simulink model "PrkgClimaActvMgr"  (R2022b)
settings: Solver=FixedStepDiscrete  StartTime=0.0  StopTime=10.0  FixedStep=0.2  RelTol=1e-3
(part 1 of 4)
settings Model: Creator=blue.ge  LastModifiedBy=blue.ge
settings DataIOCC: SaveFormat=StructureWithTime
settings OptimizationCC: DefaultParameterBehavior=Inlined  EnhancedBackFolding=on  PassReuseOutputArgsAs=Individual arguments  ZeroExternalMemoryAtStartup=off  ZeroInternalMemoryAtStartup=off  OptimizeBlockOrder=speed  DifferentSizesBufferReuse=on  OptimizationCustomize=off  EfficientTunableParamExpr=on
settings DebuggingCC: ParameterTunabilityLossMsg=error
settings ModelReferenceCC: ModelReferenceNumInstancesAllowed=Single
settings SFSimCC: MATLABDynamicMemAlloc=off
settings RTWCC: Description=AUTOSAR  SystemTargetFile=autosar.tlc  GenCodeOnly=on  TemplateMakefile=ert_default_tmf
settings CodeAppCC: ForceParamTrailComments=on  IgnoreCustomStorageClasses=off  OperatorAnnotations=on  SimulinkDataObjDesc=on  SFDataObjDesc=on  InsertBlockDesc=on
settings STFCustomTargetCC: TargetLangStandard=C99 (ISO)  MultiwordLength=256  ParMdlRefBuildCompliant=on  CompOptLevelCompliant=on  IncludeMdlTerminateFcn=off  CombineOutputUpdateFcns=on  SuppressErrorStatus=on  ERTCustomFileBanners=on  SupportNonFinite=off  SupportComplex=off  SupportContinuousTime=off  SupportNonInlinedSFcns=off  RemoveResetFunc=on  MATLABClassNameForMDSCustomization=Simulink.SoftwareTarget.ERTCustomization  AutosarCompliant=on  UseToolchainInfoCompliant=on  LookupTableObjectStructAxisOrder=2,1,3,4,...  LUTObjectStructOrderEvenSpacing=Size,Table,Breakpoints  SystemTargetFile=autosar.tlc
settings ERTTargetCC: TargetLangStandard=C99 (ISO)  ConcurrentExecutionCompliant=off  IncludeMdlTerminateFcn=off  SuppressErrorStatus=on  SupportNonFinite=off  SupportComplex=off  ModelStepFunctionPrototypeControlCompliant=off  CPPClassGenCompliant=off  AutosarCompliant=on  LookupTableObjectStructAxisOrder=2,1,3,4,...  LUTObjectStructOrderEvenSpacing=Size,Table,Breakpoints  InlinedParameterPlacement=NonHierarchical  ERTCustomFileTemplate=example_file_process.tlc

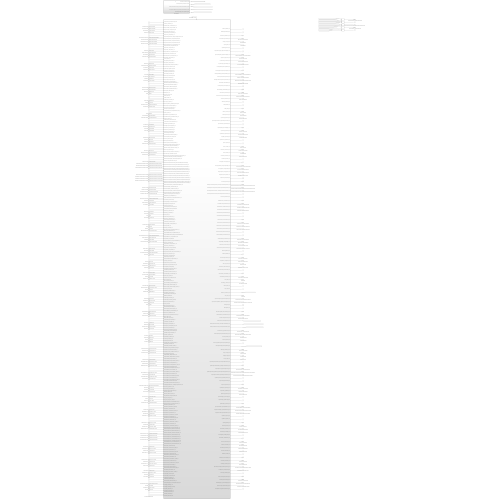
[thumbnail: Simulink's stored preview of the top-level diagram]
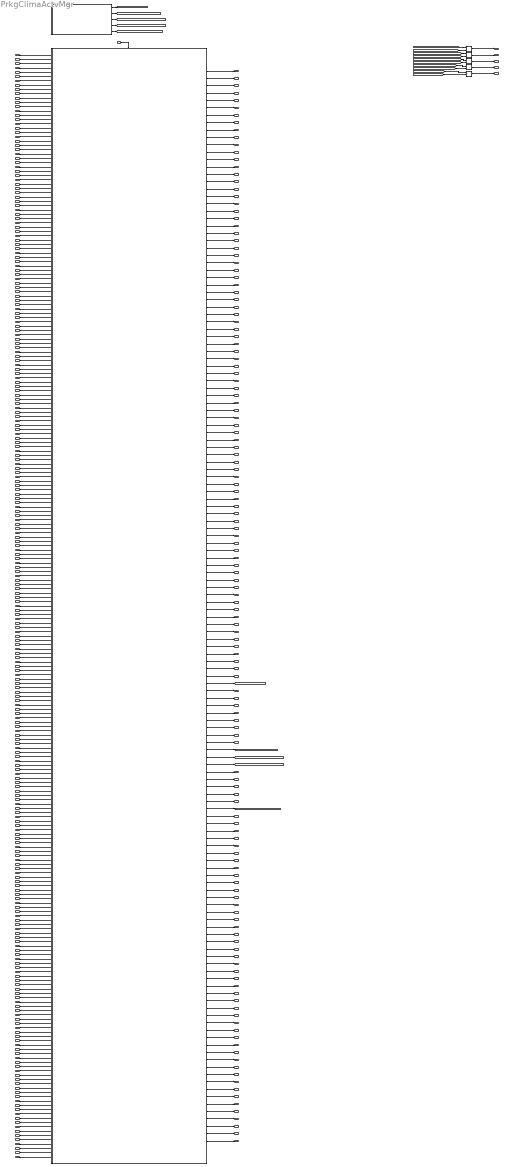
== system PrkgClimaActvMgr ==
  PrkgClimaActvMgr_Runnable : Inport  SampleTime=0.2  OutputFunctionCall=on
  ActvRqrdForPostPrkgClima_ActvRqrdForPostPrkgClima : Inport  Port=2  OutDataTypeStr=Boolean  PortDimensions=1
  CarTiIntForClima_CarTiIntForClima : Inport  Port=3  OutDataTypeStr=CarTiGlb  PortDimensions=1
  CdsClimaOnReqfromExsrc_CdsClimaOnReqfromExsrc : Inport  Port=4  OutDataTypeStr=Enum: OnOff1  PortDimensions=1
  ClimaCfgForClimaCtrlMan1_ClimaCfgForClimaCtrlMan1 : Inport  Port=5  OutDataTypeStr=Boolean  PortDimensions=1
  ClimaCfgForCmptmtClng_ClimaCfgForCmptmtClng : Inport  Port=6  OutDataTypeStr=Boolean  PortDimensions=1
  ClimaCfgForFoldCSD_ClimaCfgForFoldCSD : Inport  Port=7  OutDataTypeStr=Boolean  PortDimensions=1
  ClimaCfgForNrOfTSetg1_ClimaCfgForNrOfTSetg1 : Inport  Port=8  OutDataTypeStr=Enum: ClimaCfgForNrOfTSetg1  PortDimensions=1
  ClimaCfgForPreClng1_ClimaCfgForPreClng1 : Inport  Port=9  OutDataTypeStr=Boolean  PortDimensions=1
  ClimaCfgForSeatHeatgForRowFirstPrsnt1_ClimaCfgForSeatHeatgForRowFirstPrsnt1 : Inport  Port=10  OutDataTypeStr=Boolean  PortDimensions=1
  ClimaCfgForSeatHeatgForRowSec_ClimaCfgForSeatHeatgForRowSec : Inport  Port=11  OutDataTypeStr=Enum: CarCfgForSeatHeatgForRowSecGly  PortDimensions=1
  ClimaCfgForSeatVentnForRowFirst_ClimaCfgForSeatVentnForRowFirst : Inport  Port=12  OutDataTypeStr=Enum: CarCfgForSeatVentnForRowFirst1  PortDimensions=1
  ClimaCfgForSeatVentnForRowSec_ClimaCfgForSeatVentnForRowSec : Inport  Port=13  OutDataTypeStr=Enum: CarCfgForSeatVentnForRowSecGly  PortDimensions=1
  ClimaCfgForSteerWhlHeatgPrsnt1_ClimaCfgForSteerWhlHeatgPrsnt1 : Inport  Port=14  OutDataTypeStr=Boolean  PortDimensions=1
  ClimaCfgForSteerWhlLeSide1_ClimaCfgForSteerWhlLeSide1 : Inport  Port=15  OutDataTypeStr=Boolean  PortDimensions=1
  ClimaCfgForVehElec1_ClimaCfgForVehElec1 : Inport  Port=16  OutDataTypeStr=Boolean  PortDimensions=1
  ClimaCfgForVehHyb1_ClimaCfgForVehHyb1 : Inport  Port=17  OutDataTypeStr=Boolean  PortDimensions=1
  ClimaCfgForWindHeatrPrsnt1_ClimaCfgForWindHeatrPrsnt1 : Inport  Port=18  OutDataTypeStr=Boolean  PortDimensions=1
  ClimaOvrHeatProWarnIni_ClimaOvrHeatProWarnIni : Inport  Port=19  OutDataTypeStr=Enum: ClimaOvrheatProWarnSts  PortDimensions=1
  ClimaOvrHeatReqFromExrsc_ClimaOvrHeatReqFromExrsc : Inport  Port=20  OutDataTypeStr=Enum: PreCdngReqFromExtSrc  PortDimensions=1
  ClimaOvrHeatStrtReq_ClimaOvrHeatStrtReq : Inport  Port=21  OutDataTypeStr=Boolean  PortDimensions=1
  CmptmtClsd_CmptmtClsd : Inport  Port=22  OutDataTypeStr=Boolean  PortDimensions=1
  CSDUsgChgeOffReq_CSDUsgChgeOffReq : Inport  Port=23  OutDataTypeStr=Boolean  PortDimensions=1
  CSDUsgChgeOnReq_CSDUsgChgeOnReq : Inport  Port=24  OutDataTypeStr=Boolean  PortDimensions=1
  DefrstHeatgForPrkgClimaEnad_DefrstHeatgForPrkgClimaEnad : Inport  Port=25  OutDataTypeStr=Boolean  PortDimensions=1
  DoorOpenStsForClima_DoorOpenStsForClima : Inport  Port=26  OutDataTypeStr=Boolean  PortDimensions=1
  EgyAllwdForPrkgClima_EgyAllwdForPrkgClima : Inport  Port=27  OutDataTypeStr=Boolean  PortDimensions=1
  EgyPrioForPrkgClima_EgyPrioForPrkgClima : Inport  Port=28  OutDataTypeStr=Enum: EgyPrio  PortDimensions=1
  ErsCmdByKeyStrtReq_ErsCmdByKeyStrtReq : Inport  Port=29  OutDataTypeStr=Enum: OnOff1  PortDimensions=1
  ErsRunTimelStrtReq_ErsRunTimelStrtReq : Inport  Port=30  OutDataTypeStr=Ti39  PortDimensions=1
  FacForRtForPrkgClima_FacForRtForPrkgClima : Inport  Port=31  OutDataTypeStr=Fac30  PortDimensions=1
  FrntHvacBlowerSts_FrntHvacBlowerSts : Inport  Port=32  OutDataTypeStr=Enum: HvacFanSts  PortDimensions=1
  HeatrTypForPrkgClima_HeatrTypForPrkgClima : Inport  Port=33  OutDataTypeStr=Enum: HeatrTyp  PortDimensions=1
  HeatUpRqrdForPrkgClima_HeatUpRqrdForPrkgClima : Inport  Port=34  OutDataTypeStr=Boolean  PortDimensions=1
  HMIExtVentFanVolReqStrtReq_HMIExtVentFanVolReqStrtReq : Inport  Port=35  OutDataTypeStr=Enum: FanVolReq  PortDimensions=1
  HMIExtVentonoffReqStrtReq_HMIExtVentonoffReqStrtReq : Inport  Port=36  OutDataTypeStr=Enum: OnOffNoReq  PortDimensions=1
  HMIExtVentTSpReqStrtReq_HMIExtVentTSpReqStrtReq : Inport  Port=37  OutDataTypeStr=Enum: TSpReq  PortDimensions=1
  HMIExtVentTStsReqStrtReq_HMIExtVentTStsReqStrtReq : Inport  Port=38  OutMin=-256  OutMax=255.9  OutDataTypeStr=T2  PortDimensions=1
  HvBattForPrkgClima_HvBattForPrkgClima : Inport  Port=39  OutDataTypeStr=Boolean  PortDimensions=1
  HvBattSoc_HvBattSoc : Inport  Port=40  OutDataTypeStr=Perc7  PortDimensions=1
  HvSysCnctWoU_HvSysCnctWoU : Inport  Port=41  OutDataTypeStr=Boolean  PortDimensions=1
  HvSysWoU_HvSysWoU : Inport  Port=42  OutDataTypeStr=Boolean  PortDimensions=1
  HvSysRlySts_HvSysRlySts : Inport  Port=43  OutDataTypeStr=Bus: HvSysRlySts  PortDimensions=1  BusOutputAsStruct=on
  IntCo2MontrStrtReq_IntCo2MontrStrtReq : Inport  Port=44  OutDataTypeStr=Boolean  PortDimensions=1
  LockgCenSts_Ngcc_LockgCenSts : Inport  Port=45  OutDataTypeStr=Bus: LockgCenSts3  PortDimensions=1  BusOutputAsStruct=on
  MemReadForPrkgClimaActvMgr_MemReadForPrkgClimaActvMgr : Inport  Port=46  OutDataTypeStr=Bus: MemRecForPrkgClimaActvMgr  PortDimensions=1  BusOutputAsStruct=on
  MemReqStsForPrkgClima_MemReqStsForPrkgClima : Inport  Port=47  OutDataTypeStr=Enum: MemReqSts  PortDimensions=1
  OffForTLoForPostCdng_OffForTLoForPostCdng : Inport  Port=48  OutDataTypeStr=Boolean  PortDimensions=1
  OffRqrdForPrkgClima_OffRqrdForPrkgClima : Inport  Port=49  OutDataTypeStr=Boolean  PortDimensions=1
  PrePrkgClimaNotAvlForHvSysWoU_PrePrkgClimaNotAvlForHvSysWoU : Inport  Port=50  OutDataTypeStr=Boolean  PortDimensions=1
  PrkgCDSAvl_PrkgCDSAvl : Inport  Port=51  OutDataTypeStr=Boolean  PortDimensions=1
  PrkgClimaAllwdForBattULo_PrkgClimaAllwdForBattULo : Inport  Port=52  OutDataTypeStr=Boolean  PortDimensions=1
  PrkgClimaAllwdForSwtHeatrTyp_PrkgClimaAllwdForSwtHeatrTyp : Inport  Port=53  OutDataTypeStr=Boolean  PortDimensions=1
  PrkgHeatgActv_PrkgHeatgActv : Inport  Port=54  OutDataTypeStr=Boolean  PortDimensions=1
  PwrAllwdForHvacFanFrnt_PwrAllwdForHvacFanFrnt : Inport  Port=55  OutDataTypeStr=Pwr33  PortDimensions=1
  PwrCnsDesForHvacFanFrnt_PwrCnsDesForHvacFanFrnt : Inport  Port=56  OutDataTypeStr=Pwr33  PortDimensions=1
  RemClimaallExsrcReq_RemClimaallExsrcReq : Inport  Port=57  OutDataTypeStr=UInt32  PortDimensions=1
  RemClimaEgyExsrcReq_RemClimaEgyExsrcReq : Inport  Port=58  OutDataTypeStr=UInt32  PortDimensions=1
  RemClimaTmrStrtReq_RemClimaTmrStrtReq : Inport  Port=59  OutDataTypeStr=Ti33  PortDimensions=1
  RemStrtExtnTiStrtReq_RemStrtExtnTiStrtReq : Inport  Port=60  OutDataTypeStr=Ti39  PortDimensions=1
  RemStrtHvCtrlStrtReq_RemStrtHvCtrlStrtReq : Inport  Port=61  OutDataTypeStr=Enum: ErsCmd  PortDimensions=1
  RemVentReqStrtReq_RemVentReqStrtReq : Inport  Port=62  OutDataTypeStr=Boolean  PortDimensions=1
  RtCmftForImdtPrePrkgClima_RtCmftForImdtPrePrkgClima : Inport  Port=63  OutDataTypeStr=Ti33  PortDimensions=1
  RtCmftForPostPrkgClima_RtCmftForPostPrkgClima : Inport  Port=64  OutDataTypeStr=Ti33  PortDimensions=1
  RtCmftForPreClng_RtCmftForPreClng : Inport  Port=65  OutDataTypeStr=Ti33  PortDimensions=1
  RtSdlForPrkgClima_RtSdlForPrkgClima : Inport  Port=66  OutDataTypeStr=Ti33  PortDimensions=1
  SeatHeatForPrkgClimaEnad_SeatHeatForPrkgClimaEnad : Inport  Port=67  OutDataTypeStr=Enum: SeatHeatDurgClimaEnad  PortDimensions=1
  SteerWhlHeatgForPrkgClimaEnad_SteerWhlHeatgForPrkgClimaEnad : Inport  Port=68  OutDataTypeStr=Boolean  PortDimensions=1
  StrtRemSts1WdStrtRemSts_Ngcc_StrtRemSts1WdStrtRemSts : Inport  Port=69  OutDataTypeStr=Enum: StrtRemSts1  PortDimensions=1
  StrtRqrdForDirForPrePrkgClima_StrtRqrdForDirForPrePrkgClima : Inport  Port=70  OutDataTypeStr=Boolean  PortDimensions=1
  StrtRqrdForPreClng_StrtRqrdForPreClng : Inport  Port=71  OutDataTypeStr=Boolean  PortDimensions=1
  StrtRqrdForTmrForPrePrkgClima_StrtRqrdForTmrForPrePrkgClima : Inport  Port=72  OutDataTypeStr=Boolean  PortDimensions=1
  SuspStrtAllwdForPrkgClima_SuspStrtAllwdForPrkgClima : Inport  Port=73  OutDataTypeStr=Boolean  PortDimensions=1
  TelmBookClimaReqHmiCmptmtTSpSpclStrtReq_TelmBookClimaReqHmiCmptmtTSpSpclStrtReq : Inport  Port=74  OutDataTypeStr=Enum: NormalLowHigh  PortDimensions=1
  TelmBookClimaReqOnOffNoReqStrtReq_TelmBookClimaReqOnOffNoReqStrtReq : Inport  Port=75  OutDataTypeStr=Enum: NoReqOnOff  PortDimensions=1
  TelmBookClimaReqTempRangeStrtReq_TelmBookClimaReqTempRangeStrtReq : Inport  Port=76  OutDataTypeStr=TempRange  PortDimensions=1
  TelmBookClimaTmrStrtReq_TelmBookClimaTmrStrtReq : Inport  Port=77  OutDataTypeStr=Ti33  PortDimensions=1
  TelmBookSeatHeatClimalReqDrvHeatClimaLvlStrtReq_TelmBookSeatHeatClimalReqDrvHeatClimaLvlStrtReq : Inport  Port=78  OutDataTypeStr=Enum: TelmSeatClimaLvl  PortDimensions=1
  TelmBookSeatHeatClimalReqPassHeatClimaLvlStrtReq_TelmBookSeatHeatClimalReqPassHeatClimaLvlStrtReq : Inport  Port=79  OutDataTypeStr=Enum: TelmSeatClimaLvl  PortDimensions=1
  TelmBookSeatHeatClimalReqSecLeHeatClimaLvlStrtReq_TelmBookSeatHeatClimalReqSecLeHeatClimaLvlStrtReq : Inport  Port=80  OutDataTypeStr=Enum: TelmSeatClimaLvl  PortDimensions=1
  TelmBookSeatHeatClimalReqSecRiHeatClimaLvlStrtReq_TelmBookSeatHeatClimalReqSecRiHeatClimaLvlStrtReq : Inport  Port=81  OutDataTypeStr=Enum: TelmSeatClimaLvl  PortDimensions=1
  TelmBookSeatHeatClimalReqThrdLeHeatClimaLvlStrtReq_TelmBookSeatHeatClimalReqThrdLeHeatClimaLvlStrtReq : Inport  Port=82  OutDataTypeStr=Enum: TelmSeatClimaLvl  PortDimensions=1
  TelmBookSeatHeatClimalReqThrdRiHeatClimaLvlStrtReq_TelmBookSeatHeatClimalReqThrdRiHeatClimaLvlStrtReq : Inport  Port=83  OutDataTypeStr=Enum: TelmSeatClimaLvl  PortDimensions=1
  TelmBookSeatVentnClimalReqDrvVentnClimaLvlStrtReq_TelmBookSeatVentnClimalReqDrvVentnClimaLvlStrtReq : Inport  Port=84  OutDataTypeStr=Enum: TelmSeatClimaLvl  PortDimensions=1
  TelmBookSeatVentnClimalReqPassVentnClimaLvlStrtReq_TelmBookSeatVentnClimalReqPassVentnClimaLvlStrtReq : Inport  Port=85  OutDataTypeStr=Enum: TelmSeatClimaLvl  PortDimensions=1
  TelmBookSeatVentnClimalReqSecLeVentnClimaLvlStrtReq_TelmBookSeatVentnClimalReqSecLeVentnClimaLvlStrtReq : Inport  Port=86  OutDataTypeStr=Enum: TelmSeatClimaLvl  PortDimensions=1
  TelmBookSeatVentnClimalReqSecRiVentnClimaLvlStrtReq_TelmBookSeatVentnClimalReqSecRiVentnClimaLvlStrtReq : Inport  Port=87  OutDataTypeStr=Enum: TelmSeatClimaLvl  PortDimensions=1
  TelmBookSeatVentnClimalReqThrdLeVentnClimaLvlStrtReq_TelmBookSeatVentnClimalReqThrdLeVentnClimaLvlStrtReq : Inport  Port=88  OutDataTypeStr=Enum: TelmSeatClimaLvl  PortDimensions=1
  TelmBookSeatVentnClimalReqThrdRiVentnClimaLvlStrtReq_TelmBookSeatVentnClimalReqThrdRiVentnClimaLvlStrtReq : Inport  Port=89  OutDataTypeStr=Enum: TelmSeatClimaLvl  PortDimensions=1
  TelmBookSteerWhlHeatClimalReqStrtReq_TelmBookSteerWhlHeatClimalReqStrtReq : Inport  Port=90  OutDataTypeStr=Enum: OnOff1  PortDimensions=1
  TelmClimaExtVentnLvlStrtReq_TelmClimaExtVentnLvlStrtReq : Inport  Port=91  OutDataTypeStr=Enum: HmiHvacFanLvl  PortDimensions=1
  TelmClimaExtVentnReqStrtReq_TelmClimaExtVentnReqStrtReq : Inport  Port=92  OutDataTypeStr=Enum: OnOffNoReq  PortDimensions=1
  TelmClimaExtVentnTempRangeStrtReq_TelmClimaExtVentnTempRangeStrtReq : Inport  Port=93  OutMin=-256  OutMax=255.9  OutDataTypeStr=T2  PortDimensions=1
  TelmClimaExtVentnTSpSpclStrtReq_TelmClimaExtVentnTSpSpclStrtReq : Inport  Port=94  OutDataTypeStr=Enum: HmiCmptmtTSpSpcl  PortDimensions=1
  UpdRtTotForTmrForPrePrkgClima_UpdRtTotForTmrForPrePrkgClima : Inport  Port=95  OutDataTypeStr=Boolean  PortDimensions=1
  VehModMngtGlbSafe1_Ngcc_VehModMngtGlbSafe1 : Inport  Port=96  OutDataTypeStr=Bus: VehModMngtGlbSafe1  PortDimensions=1  BusOutputAsStruct=on
  VFCBodyPreClimatisationHmiHumCtrl_VFCBodyPreClimatisationHmiHumCtrl : Inport  Port=97  OutDataTypeStr=Boolean  PortDimensions=1
  VFCHmiClimaCtrlAct_VFCHmiClimaCtrlAct : Inport  Port=98  OutDataTypeStr=Enum: OnOff1  PortDimensions=1
  VFCPrkDrvgClimabyFragAct_VFCPrkDrvgClimabyFragAct : Inport  Port=99  OutDataTypeStr=Boolean  PortDimensions=1
  WarnForEgyAllwdLimd_WarnForEgyAllwdLimd : Inport  Port=100  OutDataTypeStr=Boolean  PortDimensions=1
  WarnForHybWoU_WarnForHybWoU : Inport  Port=101  OutDataTypeStr=Boolean  PortDimensions=1
  WarnForPreCdngFctNotAvl_WarnForPreCdngFctNotAvl : Inport  Port=102  OutDataTypeStr=Boolean  PortDimensions=1
  WarnForPrkgClimaNotAllwd_WarnForPrkgClimaNotAllwd : Inport  Port=103  OutDataTypeStr=Enum: ClimaWarn  PortDimensions=1
  WindIceDetdFrnt_Ngcc_WindIceDetdFrnt : Inport  Port=104  OutDataTypeStr=Enum: OnOff1  PortDimensions=1
  WindIceDetdRe_Ngcc_WindIceDetdRe : Inport  Port=105  OutDataTypeStr=Enum: OnOff1  PortDimensions=1
  AirCdnAvl_AirCdnAvl : Inport  Port=106  OutDataTypeStr=Boolean  PortDimensions=1
  AmbTFildForClimaNgcc_AmbTFildForClima : Inport  Port=107  OutDataTypeStr=T30  PortDimensions=1
  OffRqrdForRemClima_OffRqrdForRemClima : Inport  Port=108  OutDataTypeStr=Boolean  PortDimensions=1
  RemClimaTempStrtReq_RemClimaTempStrtReq : Inport  Port=109  OutMin=-256  OutMax=255.9  OutDataTypeStr=T2  PortDimensions=1
  VFCAutDemstOperSts_VFCAutDemstOperSts : Inport  Port=110  OutDataTypeStr=Boolean  PortDimensions=1
  VFCPrkDrvgClimabyPM25_VFCPrkDrvgClimabyPM25 : Inport  Port=111  OutDataTypeStr=Boolean  PortDimensions=1
  AirCdnAvlRe_AirCdnAvlRe : Inport  Port=112  OutDataTypeStr=Boolean  PortDimensions=1
  AirSrcStsFbNgcc_AirSrcStsFbNgcc : Inport  Port=113  OutMin=0  OutMax=3  OutDataTypeStr=UInt2  PortDimensions=1
  BookTrvlClimaActvReqfromExsrc_BookTrvlClimaActvReqfromExsrc : Inport  Port=114  OutDataTypeStr=Enum: OnOffNoReq  PortDimensions=1
  CCMWakeupFunction_CCMWakeupFunction : Inport  Port=115  OutDataTypeStr=UInt8  PortDimensions=1
  ClimaCfgFor3RDROWSEATHEAT_ClimaCfgFor3RDROWSEATHEAT : Inport  Port=116  OutDataTypeStr=UInt8  PortDimensions=1
  ClimaCfgFor3RDROWSEATVENTILATED_ClimaCfgFor3RDROWSEATVENTILATED : Inport  Port=117  OutDataTypeStr=UInt8  PortDimensions=1
  ClimaCfgForAirMtrlSnsrPrsnt1_ClimaCfgForAirMtrlSnsrPrsnt1 : Inport  Port=118  OutDataTypeStr=Enum: CarCfgForAirMtrlSnsrGly  PortDimensions=1
  ClimaCfgForBRAND_ClimaCfgForBRAND : Inport  Port=119  OutDataTypeStr=Enum: ClimaCfgForBRAND  PortDimensions=1
  ClimaCfgForDrvrSeatVentWthSmf2_ClimaCfgForDrvrSeatVentWthSmf2 : Inport  Port=120  OutDataTypeStr=UInt8  PortDimensions=1
  ClimaCfgForErev1_ClimaCfgForErev1 : Inport  Port=121  OutDataTypeStr=Boolean  PortDimensions=1
  ClimaCfgForHvacRePrsnt1_ClimaCfgForHvacRePrsnt1 : Inport  Port=122  OutDataTypeStr=Enum: CarCfgForHvacRe  PortDimensions=1
  ClimaCfgForHvacTyp_ClimaCfgForHvacTyp : Inport  Port=123  OutDataTypeStr=Enum: CarCfgForHvacTyp2  PortDimensions=1
  ClimaCfgForLifeDetection_ClimaCfgForLifeDetection : Inport  Port=124  OutDataTypeStr=Enum: LifeDetection  PortDimensions=1
  ClimaCfgForMARKET_ClimaCfgForMARKET : Inport  Port=125  OutDataTypeStr=UInt8  PortDimensions=1
  ClimaCfgForPassSeatVentWthSmf2_ClimaCfgForPassSeatVentWthSmf2 : Inport  Port=126  OutDataTypeStr=UInt8  PortDimensions=1
  ClimaCfgForPrpsnTyp_ClimaCfgForPrpsnTyp : Inport  Port=127  OutDataTypeStr=Enum: CarCfgForPrpsnTypGly  PortDimensions=1
  ClimaCfgForRoofHatch_ClimaCfgForRoofHatch : Inport  Port=128  OutDataTypeStr=Enum: CarCfgForRoofHatch  PortDimensions=1
  ClimaCfgForVehTyp_ClimaCfgForVehTyp : Inport  Port=129  OutDataTypeStr=Enum: CarCfgForVehTyp3  PortDimensions=1
  ClimaCfgReqVersionReplace_ClimaCfgReqVersionReplace : Inport  Port=130  OutDataTypeStr=UInt8  PortDimensions=1
  CmptmtClngActv_CmptmtClngActv : Inport  Port=131  OutDataTypeStr=Enum: CmptmtClngActv  PortDimensions=1
  CmptmtOpenStsForClima_CmptmtOpenStsForClima : Inport  Port=132  OutDataTypeStr=Boolean  PortDimensions=1
  DoorStsWithOutTrForClima_DoorStsWithOutTrForClima : Inport  Port=133  OutDataTypeStr=Boolean  PortDimensions=1
  DrvrSeatHeatgLvlSts_DrvrSeatHeatgLvlSts : Inport  Port=134  OutDataTypeStr=Enum: SeatClimaLvl  PortDimensions=1
  DrvrSeatHeatgLvlSts_DrvrSeatHeatgLvlSts_IsUpdated : Inport  Port=135  OutDataTypeStr=boolean  PortDimensions=1
  DrvrSeatVentnLvlSts_DrvrSeatVentnLvlSts : Inport  Port=136  OutDataTypeStr=Enum: SeatClimaLvl  PortDimensions=1
  DrvrSeatVentnLvlSts_DrvrSeatVentnLvlSts_IsUpdated : Inport  Port=137  OutDataTypeStr=boolean  PortDimensions=1
  DrvrSeatVentnLvlSts2Ngcc_DrvrSeatVentnLvlSts2Ngcc : Inport  Port=138  OutDataTypeStr=Enum: SeatClimaLvl  PortDimensions=1
  EngSt1WdSts_Ngcc_EngSt1WdSts : Inport  Port=139  OutDataTypeStr=Enum: EngSt1  PortDimensions=1
  ErsCmdByKeyFromExsrc_ErsCmdByKeyFromExsrc : Inport  Port=140  OutDataTypeStr=Enum: ErsCmd  PortDimensions=1
  GearLvrIndcn_GearLvrIndcn : Inport  Port=141  OutDataTypeStr=Enum: GearLvrIndcn2  PortDimensions=1
  GearLvrIndcn_GearLvrIndcn_IsUpdated : Inport  Port=142  OutDataTypeStr=boolean  PortDimensions=1
  HighTempCleanHmiReqNgcc_HighTempCleanHmiReqNgcc : Inport  Port=143  OutDataTypeStr=Enum: NoReqOnOff  PortDimensions=1
  HMIAirCdnrAutDrySetNgcc_HMIAirCdnrAutDrySetNgcc : Inport  Port=144  OutDataTypeStr=Enum: OnOff1  PortDimensions=1
  HMIAirCdnrAutDrySetNgcc_HMIAirCdnrAutDrySetNgcc_IsUpdated : Inport  Port=145  OutDataTypeStr=boolean  PortDimensions=1
  HmiCmptmtTSp_HmiCmptmtTSp : Inport  Port=146  OutDataTypeStr=Bus: HmiCmptmtTSp  PortDimensions=1  BusOutputAsStruct=on
  HMILBRACStrtSetNgcc_HMILBRACStrtSetNgcc : Inport  Port=147  OutDataTypeStr=Enum: OnOff1  PortDimensions=1
  HmiParkVentSetNgcc_HmiParkVentSetNgcc : Inport  Port=148  OutDataTypeStr=Enum: OnOff1  PortDimensions=1
  HmiParkVentTmrSetNgcc_HmiParkVentTmrSetNgcc : Inport  Port=149  OutMin=0  OutMax=31  OutDataTypeStr=TimeHr5  PortDimensions=1
  HvBattMntnActv_HvBattMntnActv : Inport  Port=150  OutDataTypeStr=Boolean  PortDimensions=1
  HvCooltHeatrStsSig_HvCooltHeatrStsSig : Inport  Port=151  OutDataTypeStr=Enum: HvCooltHeatrSts  PortDimensions=1
  HvCooltHeatrStsSig_HvCooltHeatrStsSig_IsUpdated : Inport  Port=152  OutDataTypeStr=boolean  PortDimensions=1
  IntPm25VluFrmClima_IntPm25VluFrmClima : Inport  Port=153  OutDataTypeStr=UInt10  PortDimensions=1
  LDACState_LDACState : Inport  Port=154  OutDataTypeStr=Boolean  PortDimensions=1
  MemBookTrvlClimaTi_MemBookTrvlClimaTi : Inport  Port=155  OutDataTypeStr=Mins2  PortDimensions=1
  MemReadForBlwAftRun_MemReadForBlwAftRun : Inport  Port=156  OutDataTypeStr=Bus: MemRecForBlwAftRun  PortDimensions=1  BusOutputAsStruct=on
  MemReadForHighTempCln_MemReadForHighTempCln : Inport  Port=157  OutDataTypeStr=Bus: MemRecForHighTempCln  PortDimensions=1  BusOutputAsStruct=on
  MemReadForHMISetEngReq_MemReadForHMISetEngReq : Inport  Port=158  OutDataTypeStr=Bus: MemRecForHMISetEngReq  PortDimensions=1  BusOutputAsStruct=on
  MemReadForParkVent_MemReadForParkVent : Inport  Port=159  OutDataTypeStr=Bus: MemRecForParkVent  PortDimensions=1  BusOutputAsStruct=on
  MemReadForParkVentActvCnt_MemReadForParkVentActvCnt : Inport  Port=160  OutDataTypeStr=Bus: MemRecForParkVentCnt  PortDimensions=1  BusOutputAsStruct=on
  OnBdChrgrSt_OnBdChrgrSt : Inport  Port=161  OutDataTypeStr=Enum: ChrgrSts1  PortDimensions=1
  OnBdChrgrSt_OnBdChrgrSt_IsUpdated : Inport  Port=162  OutDataTypeStr=boolean  PortDimensions=1
  OxygenSplyAvlStsNgcc_OxygenSplyAvlStsNgcc : Inport  Port=163  OutDataTypeStr=Enum: OxygenSplyAvlSts  PortDimensions=1
  OxygenSplyErrNgcc_OxygenSplyErrNgcc : Inport  Port=164  OutDataTypeStr=Enum: Err1  PortDimensions=1
  PassSeatHeatgLvlSts_PassSeatHeatgLvlSts : Inport  Port=165  OutDataTypeStr=Enum: SeatClimaLvl  PortDimensions=1
  PassSeatHeatgLvlSts_PassSeatHeatgLvlSts_IsUpdated : Inport  Port=166  OutDataTypeStr=boolean  PortDimensions=1
  PassSeatVentnLvlSts_PassSeatVentnLvlSts : Inport  Port=167  OutDataTypeStr=Enum: SeatClimaLvl  PortDimensions=1
  PassSeatVentnLvlSts_PassSeatVentnLvlSts_IsUpdated : Inport  Port=168  OutDataTypeStr=boolean  PortDimensions=1
  PassSeatVentnLvlSts2Ngcc_PassSeatVentnLvlSts2Ngcc : Inport  Port=169  OutDataTypeStr=Enum: SeatClimaLvl  PortDimensions=1
  PrkgClimaReqExcpSeat_PrkgClimaReqExcpSeat : Inport  Port=170  OutDataTypeStr=Boolean  PortDimensions=1
  PrkgClimaReqPrio_PrkgClimaReqPrio : Inport  Port=171  OutDataTypeStr=Boolean  PortDimensions=1
  PrkgOGMAirSrcReq_PrkgOGMAirSrcReq : Inport  Port=172  OutDataTypeStr=Boolean  PortDimensions=1
  PrvntWinActvReq_PrvntWinActvReq : Inport  Port=173  OutDataTypeStr=Boolean  PortDimensions=1
  ReHvacBlowerSts_ReHvacBlowerSts : Inport  Port=174  OutDataTypeStr=Enum: HvacFanSts  PortDimensions=1
  RemClimaEgyExsrcReqPlus_RemClimaEgyExsrcReqPlus : Inport  Port=175  OutDataTypeStr=UInt32  PortDimensions=1
  RemClimaHVReqFb_RemClimaHVReqFb : Inport  Port=176  OutDataTypeStr=Enum: HVReqFb  PortDimensions=1
  RemClimaHVReqFb_RemClimaHVReqFb_IsUpdated : Inport  Port=177  OutDataTypeStr=boolean  PortDimensions=1
  RemClimaWarnForCmptmtClng_RemClimaWarnForCmptmtClng : Inport  Port=178  OutDataTypeStr=Enum: ClimaWarn  PortDimensions=1
  RemClimaWarnForOpenGlb_RemClimaWarnForOpenGlb : Inport  Port=179  OutDataTypeStr=Enum: ClimaWarn  PortDimensions=1
  RemClimaWarnForUnLckClima_RemClimaWarnForUnLckClima : Inport  Port=180  OutDataTypeStr=Enum: ClimaWarn  PortDimensions=1
  RemOxygenSplyReq_RemOxygenSplyReq : Inport  Port=181  OutDataTypeStr=Boolean  PortDimensions=1
  SeatHeatgLvlStsRowSecLe_SeatHeatgLvlStsRowSecLe : Inport  Port=182  OutDataTypeStr=Enum: SeatClimaLvl  PortDimensions=1
  SeatHeatgLvlStsRowSecLe_SeatHeatgLvlStsRowSecLe_IsUpdated : Inport  Port=183  OutDataTypeStr=boolean  PortDimensions=1
  SeatHeatgLvlStsRowSecRi_SeatHeatgLvlStsRowSecRi : Inport  Port=184  OutDataTypeStr=Enum: SeatClimaLvl  PortDimensions=1
  SeatHeatgLvlStsRowSecRi_SeatHeatgLvlStsRowSecRi_IsUpdated : Inport  Port=185  OutDataTypeStr=boolean  PortDimensions=1
  SeatHeatgLvlStsRowThrdLe_SeatHeatgLvlStsRowThrdLe : Inport  Port=186  OutDataTypeStr=Enum: SeatClimaLvl  PortDimensions=1
  SeatHeatgLvlStsRowThrdLe_SeatHeatgLvlStsRowThrdLe_IsUpdated : Inport  Port=187  OutDataTypeStr=boolean  PortDimensions=1
  SeatHeatgLvlStsRowThrdRi_SeatHeatgLvlStsRowThrdRi : Inport  Port=188  OutDataTypeStr=Enum: SeatClimaLvl  PortDimensions=1
  SeatHeatgLvlStsRowThrdRi_SeatHeatgLvlStsRowThrdRi_IsUpdated : Inport  Port=189  OutDataTypeStr=boolean  PortDimensions=1
  SeatVentnLvlStsRowSecLe_SeatVentnLvlStsRowSecLe : Inport  Port=190  OutDataTypeStr=Enum: SeatClimaLvl  PortDimensions=1
  SeatVentnLvlStsRowSecLe_SeatVentnLvlStsRowSecLe_IsUpdated : Inport  Port=191  OutDataTypeStr=boolean  PortDimensions=1
  SeatVentnLvlStsRowSecRi_SeatVentnLvlStsRowSecRi : Inport  Port=192  OutDataTypeStr=Enum: SeatClimaLvl  PortDimensions=1
  SeatVentnLvlStsRowSecRi_SeatVentnLvlStsRowSecRi_IsUpdated : Inport  Port=193  OutDataTypeStr=boolean  PortDimensions=1
  SeatVentnLvlStsRowThrdLe_SeatVentnLvlStsRowThrdLe : Inport  Port=194  OutDataTypeStr=Enum: SeatClimaLvl  PortDimensions=1
  SeatVentnLvlStsRowThrdLe_SeatVentnLvlStsRowThrdLe_IsUpdated : Inport  Port=195  OutDataTypeStr=boolean  PortDimensions=1
  SeatVentnLvlStsRowThrdRi_SeatVentnLvlStsRowThrdRi : Inport  Port=196  OutDataTypeStr=Enum: SeatClimaLvl  PortDimensions=1
  SeatVentnLvlStsRowThrdRi_SeatVentnLvlStsRowThrdRi_IsUpdated : Inport  Port=197  OutDataTypeStr=boolean  PortDimensions=1
  SteerWhlHeatgDurgClimaEnadFromExsrc_SteerWhlHeatgDurgClimaEnadFromExsrc : Inport  Port=198  OutDataTypeStr=Enum: OnOffNoReq  PortDimensions=1
  SunRoofPosnSts_Ngcc_SunRoofPosnSts : Inport  Port=199  OutDataTypeStr=Enum: WinAndRoofAndCurtPosnTyp  PortDimensions=1
  TcsOvrheatdProtnReq_TcsOvrheatdProtnReq : Inport  Port=200  OutDataTypeStr=Boolean  PortDimensions=1
  TelmClimaTmrFromExtSrc_TelmClimaTmrFromExtSrc : Inport  Port=201  OutDataTypeStr=Ti33  PortDimensions=1
  TelmDefrostTmr_TelmDefrostTmr : Inport  Port=202  OutDataTypeStr=TimeDesForSWDL  PortDimensions=1
  TelmDefrostTmr_TelmDefrostTmr_IsUpdated : Inport  Port=203  OutDataTypeStr=boolean  PortDimensions=1
  TelmOxygenReqFromExSrc_TelmOxygenReqFromExSrc : Inport  Port=204  OutDataTypeStr=Enum: OnOffNoReq  PortDimensions=1
  TelmOxygenTmr_TelmOxygenTmr : Inport  Port=205  OutDataTypeStr=TimeDesForSWDL  PortDimensions=1
  TelmOxygenTmr_TelmOxygenTmr_IsUpdated : Inport  Port=206  OutDataTypeStr=boolean  PortDimensions=1
  TelmSeatDrvrHeatReqFromExsrc_TelmSeatDrvrHeatReqFromExsrc : Inport  Port=207  OutDataTypeStr=Enum: TelmSeatClimaLvlExsrc  PortDimensions=1
  TelmSeatDrvVentnReqFromExsrc_TelmSeatDrvVentnReqFromExsrc : Inport  Port=208  OutDataTypeStr=Enum: TelmSeatClimaLvlExsrc  PortDimensions=1
  TelmSeatHeatDrvrTmr_TelmSeatHeatDrvrTmr : Inport  Port=209  OutDataTypeStr=TimeDesForSWDL  PortDimensions=1
  TelmSeatHeatDrvrTmr_TelmSeatHeatDrvrTmr_IsUpdated : Inport  Port=210  OutDataTypeStr=boolean  PortDimensions=1
  TelmSeatHeatPassTmr_TelmSeatHeatPassTmr : Inport  Port=211  OutDataTypeStr=TimeDesForSWDL  PortDimensions=1
  TelmSeatHeatPassTmr_TelmSeatHeatPassTmr_IsUpdated : Inport  Port=212  OutDataTypeStr=boolean  PortDimensions=1
  TelmSeatHeatSecLeTmr_TelmSeatHeatSecLeTmr : Inport  Port=213  OutDataTypeStr=TimeDesForSWDL  PortDimensions=1
  TelmSeatHeatSecLeTmr_TelmSeatHeatSecLeTmr_IsUpdated : Inport  Port=214  OutDataTypeStr=boolean  PortDimensions=1
  TelmSeatHeatSecRiTmr_TelmSeatHeatSecRiTmr : Inport  Port=215  OutDataTypeStr=TimeDesForSWDL  PortDimensions=1
  TelmSeatHeatSecRiTmr_TelmSeatHeatSecRiTmr_IsUpdated : Inport  Port=216  OutDataTypeStr=boolean  PortDimensions=1
  TelmSeatHeatThrdLeTmr_TelmSeatHeatThrdLeTmr : Inport  Port=217  OutDataTypeStr=TimeDesForSWDL  PortDimensions=1
  TelmSeatHeatThrdLeTmr_TelmSeatHeatThrdLeTmr_IsUpdated : Inport  Port=218  OutDataTypeStr=boolean  PortDimensions=1
  TelmSeatHeatThrdRiTmr_TelmSeatHeatThrdRiTmr : Inport  Port=219  OutDataTypeStr=TimeDesForSWDL  PortDimensions=1
  TelmSeatHeatThrdRiTmr_TelmSeatHeatThrdRiTmr_IsUpdated : Inport  Port=220  OutDataTypeStr=boolean  PortDimensions=1
  TelmSeatPassHeatReqFromExsrc_TelmSeatPassHeatReqFromExsrc : Inport  Port=221  OutDataTypeStr=Enum: TelmSeatClimaLvlExsrc  PortDimensions=1
  TelmSeatPassVentnReqFromExsrc_TelmSeatPassVentnReqFromExsrc : Inport  Port=222  OutDataTypeStr=Enum: TelmSeatClimaLvlExsrc  PortDimensions=1
  TelmSeatSecLeHeatReqFromExsrc_TelmSeatSecLeHeatReqFromExsrc : Inport  Port=223  OutDataTypeStr=Enum: TelmSeatClimaLvlExsrc  PortDimensions=1
  TelmSeatSecLeVentnReqFromExsrc_TelmSeatSecLeVentnReqFromExsrc : Inport  Port=224  OutDataTypeStr=Enum: TelmSeatClimaLvlExsrc  PortDimensions=1
  TelmSeatSecRiHeatReqFromExsrc_TelmSeatSecRiHeatReqFromExsrc : Inport  Port=225  OutDataTypeStr=Enum: TelmSeatClimaLvlExsrc  PortDimensions=1
  TelmSeatSecRiVentnReqFromExsrc_TelmSeatSecRiVentnReqFromExsrc : Inport  Port=226  OutDataTypeStr=Enum: TelmSeatClimaLvlExsrc  PortDimensions=1
  TelmSeatThrdLeHeatReqFromExsrc_TelmSeatThrdLeHeatReqFromExsrc : Inport  Port=227  OutDataTypeStr=Enum: TelmSeatClimaLvlExsrc  PortDimensions=1
  TelmSeatThrdLeVentnReqFromExsrc_TelmSeatThrdLeVentnReqFromExsrc : Inport  Port=228  OutDataTypeStr=Enum: TelmSeatClimaLvlExsrc  PortDimensions=1
  TelmSeatThrdRiHeatReqFromExsrc_TelmSeatThrdRiHeatReqFromExsrc : Inport  Port=229  OutDataTypeStr=Enum: TelmSeatClimaLvlExsrc  PortDimensions=1
  TelmSeatThrdRiVentnReqFromExsrc_TelmSeatThrdRiVentnReqFromExsrc : Inport  Port=230  OutDataTypeStr=Enum: TelmSeatClimaLvlExsrc  PortDimensions=1
  TelmSeatVentnDrvrTmr_TelmSeatVentnDrvrTmr : Inport  Port=231  OutDataTypeStr=TimeDesForSWDL  PortDimensions=1
  TelmSeatVentnDrvrTmr_TelmSeatVentnDrvrTmr_IsUpdated : Inport  Port=232  OutDataTypeStr=boolean  PortDimensions=1
  TelmSeatVentnPassTmr_TelmSeatVentnPassTmr : Inport  Port=233  OutDataTypeStr=TimeDesForSWDL  PortDimensions=1
  TelmSeatVentnPassTmr_TelmSeatVentnPassTmr_IsUpdated : Inport  Port=234  OutDataTypeStr=boolean  PortDimensions=1
  TelmSeatVentnSecLeTmr_TelmSeatVentnSecLeTmr : Inport  Port=235  OutDataTypeStr=TimeDesForSWDL  PortDimensions=1
  TelmSeatVentnSecLeTmr_TelmSeatVentnSecLeTmr_IsUpdated : Inport  Port=236  OutDataTypeStr=boolean  PortDimensions=1
  TelmSeatVentnSecRiTmr_TelmSeatVentnSecRiTmr : Inport  Port=237  OutDataTypeStr=TimeDesForSWDL  PortDimensions=1
  TelmSeatVentnSecRiTmr_TelmSeatVentnSecRiTmr_IsUpdated : Inport  Port=238  OutDataTypeStr=boolean  PortDimensions=1
  TelmSeatVentnThrdLeTmr_TelmSeatVentnThrdLeTmr : Inport  Port=239  OutDataTypeStr=TimeDesForSWDL  PortDimensions=1
  TelmSeatVentnThrdLeTmr_TelmSeatVentnThrdLeTmr_IsUpdated : Inport  Port=240  OutDataTypeStr=boolean  PortDimensions=1
  TelmSeatVentnThrdReq_TelmSeatVentnThrdReq : Inport  Port=241  OutDataTypeStr=UInt32  PortDimensions=1
  TelmSeatVentnThrdRiTmr_TelmSeatVentnThrdRiTmr : Inport  Port=242  OutDataTypeStr=TimeDesForSWDL  PortDimensions=1
  TelmSeatVentnThrdRiTmr_TelmSeatVentnThrdRiTmr_IsUpdated : Inport  Port=243  OutDataTypeStr=boolean  PortDimensions=1
  TelmSteerWhlHeatTmr_TelmSteerWhlHeatTmr : Inport  Port=244  OutDataTypeStr=TimeDesForSWDL  PortDimensions=1
  TelmSteerWhlHeatTmr_TelmSteerWhlHeatTmr_IsUpdated : Inport  Port=245  OutDataTypeStr=boolean  PortDimensions=1
  UnLckClimaActvReq_UnLckClimaActvReq : Inport  Port=246  OutDataTypeStr=Enum: UnLckCoolActvLvl  PortDimensions=1
  UnLckClimaActvReqSt_UnLckClimaActvReqSt : Inport  Port=247  OutDataTypeStr=Boolean  PortDimensions=1
  UnLckHeatActvReq_UnLckHeatActvReq : Inport  Port=248  OutDataTypeStr=Enum: UnLckHeatActvLvl  PortDimensions=1
  UnLckHeatActvReqSt_UnLckHeatActvReqSt : Inport  Port=249  OutDataTypeStr=Boolean  PortDimensions=1
  VentnForWinOpenGlbActv_VentnForWinOpenGlbActv : Inport  Port=250  OutDataTypeStr=Boolean  PortDimensions=1
  VFCPrkDrvgClimabyOxygenSplyCtrl_VFCPrkDrvgClimabyOxygenSplyCtrl : Inport  Port=251  OutDataTypeStr=Boolean  PortDimensions=1
  WarnLvlMsgNgcc_WarnLvlMsgNgcc : Inport  Port=252  OutDataTypeStr=Enum: WarnLvl  PortDimensions=1
  WarnLvlMsgNgcc_WarnLvlMsgNgcc_IsUpdated : Inport  Port=253  OutDataTypeStr=boolean  PortDimensions=1
  WinOpenGlbReq_WinOpenGlbReq : Inport  Port=254  OutDataTypeStr=Boolean  PortDimensions=1
  WinPosnStsAtReLe_WinPosnStsAtReLe : Inport  Port=255  OutDataTypeStr=Enum: WinAndRoofAndCurtPosnTyp  PortDimensions=1
  WinPosnStsAtReRi_WinPosnStsAtReRi : Inport  Port=256  OutDataTypeStr=Enum: WinAndRoofAndCurtPosnTyp  PortDimensions=1
  WinPosnStsDrv_WinPosnStsAtDrv : Inport  Port=257  OutDataTypeStr=Enum: WinAndRoofAndCurtPosnTyp  PortDimensions=1
  WinPosnStsPass_WinPosnStsAtPass : Inport  Port=258  OutDataTypeStr=Enum: WinAndRoofAndCurtPosnTyp  PortDimensions=1
  Co2WarnForTelm_Co2WarnForTelm_merge : Merge
  ExtVentTempStsFrmClimaExtVentTemprSts_ExtVentTempStsFrmClimaExtVentTemprSts_merge : Merge
  ExtVentTempStsFrmClimaExtVentTemprVal_ExtVentTempStsFrmClimaExtVentTemprVal_merge : Merge
  ExtVentTempStsFrmClima_ExtVentTempStsFrmClima_merge : Merge
  MemWrForPrkgClimaActvMgr_MemWrForPrkgClimaActvMgr_merge : Merge
  PrkgClimaActvMgr_Init : Subsystem
  PrkgClimaActvMgr_Runnable_sys : Subsystem
  Co2WarnForTelm_Co2WarnForTelm : Outport  OutDataTypeStr=Enum: OnOff1  PortDimensions=1
  ExtVentTempStsFrmClima_ExtVentTempStsFrmClima : Outport  Port=2  OutDataTypeStr=Bus: ExtVentTempStsFrmClimaGrp  PortDimensions=1  BusOutputAsStruct=on
  ExtVentTempStsFrmClimaExtVentTemprSts_ExtVentTempStsFrmClimaExtVentTemprSts : Outport  Port=3  OutDataTypeStr=Enum: ExtVentTemprSts  PortDimensions=1
  ExtVentTempStsFrmClimaExtVentTemprVal_ExtVentTempStsFrmClimaExtVentTemprVal : Outport  Port=4  OutDataTypeStr=Temp5  PortDimensions=1
  MemWrForPrkgClimaActvMgr_MemWrForPrkgClimaActvMgr : Outport  Port=5  OutDataTypeStr=Bus: MemRecForPrkgClimaActvMgr  PortDimensions=1  BusOutputAsStruct=on
  BlwAftRunAct_BlwAftRunAct : Outport  Port=6  OutDataTypeStr=Boolean  PortDimensions=1
  BlwAftRunReqRe_BlwAftRunReqRe : Outport  Port=7  OutDataTypeStr=Boolean  PortDimensions=1
  BlwAftRunWkTime_BlwAftRunWkTime : Outport  Port=8  OutDataTypeStr=UInt8  PortDimensions=1
  BookTrvlClimaFnshd_BookTrvlClimaFnshd : Outport  Port=9  OutDataTypeStr=Boolean  PortDimensions=1
  DsChrgClima_DsChrgClima : Outport  Port=10  OutDataTypeStr=Boolean  PortDimensions=1
  HghClnAct_HghClnAct : Outport  Port=11  OutDataTypeStr=Boolean  PortDimensions=1
  HghClnWkTime_HghClnWkTime : Outport  Port=12  OutDataTypeStr=UInt8  PortDimensions=1
  HighTempCleanRunningStsNgcc_HighTempCleanRunningStsNgcc : Outport  Port=13  OutDataTypeStr=Enum: OnOffNoReq  PortDimensions=1
  HighTempCleanSettingStsNgcc_HighTempCleanSettingStsNgcc : Outport  Port=14  OutDataTypeStr=Enum: OnOffNoReq  PortDimensions=1
  HighTempClnActv_HighTempClnActv : Outport  Port=15  OutDataTypeStr=UInt8  PortDimensions=1
  KeyClimaActvFnshd_KeyClimaActvFnshd : Outport  Port=16  OutDataTypeStr=Boolean  PortDimensions=1
  KeySynchrnztnLclNgcc_KeySynchrnztnLclNgcc : Outport  Port=17  OutDataTypeStr=Bus: RemSynchrnztnLcl  PortDimensions=1  BusOutputAsStruct=on